simulink library "lib_all"  (Simulink 7.6)
settings: Solver=ode45  SolverMode=SingleTasking  StartTime=0.0  StopTime=10.0  RelTol=1e-3
values from neighbouring files (symbols this model uses):
  map_lib_03 = [6.8679 7.0159 7.4571 8.1849 9.1892 10.456 11.97 13.71 15.655 17.781 20.063 22.474 ... (803 elements, 73x11)]    [scr_simdata.mat]    (also in scr_simdata_all.mat)
  map_lib_06 = [2.5784 2.6727 2.9588 3.4453 4.1444 5.068 6.226 7.6242 9.2634 11.139 13.24 15.552 ... (803 elements, 73x11)]    [scr_simdata.mat]    (also in scr_simdata_all.mat)
  map_lib_09 = [0.0025784 0.0026727 0.0029588 0.0034453 0.0041444 0.005068 0.006226 0.0076242 0.0092634 0.011139 0.01324 0.015552 ... (803 elements, 73x11)]    [scr_simdata.mat]    (also in scr_simdata_all.mat)
  map_lib_10 = [2.74 2.75 2.76 2.77 2.78 2.79 2.8 2.81 2.82 2.83 2.84 2.85 ... (81 elements, 1x81)]    [scr_simdata.mat]    (also in scr_simdata_all.mat)
  map_lib_11 = [1.1983 1.2027 1.207 1.2113 1.2156 1.2198 1.2241 1.2283 1.2326 1.2368 1.241 1.2451 ... (81 elements, 1x81)]    [scr_simdata.mat]    (also in scr_simdata_all.mat)
  map_lib_12 = [2.74 2.75 2.76 2.77 2.78 2.79 2.8 2.81 2.82 2.83 2.84 2.85 ... (81 elements, 1x81)]    [scr_simdata.mat]    (also in scr_simdata_all.mat)
  map_lib_13 = [1.74 1.75 1.76 1.77 1.78 1.79 1.8 1.81 1.82 1.83 1.84 1.85 ... (81 elements, 1x81)]    [scr_simdata.mat]    (also in scr_simdata_all.mat)
  map_lib_16 = [0.00048828125 1.6159 1.8962 2.0821 2.2251 2.3426 2.4433 2.5318 2.611 2.683 2.749 2.8101 ... (1419 elements, 129x11)]    [scr_simdata.mat]    (also in scr_simdata_all.mat)
  map_mr2_01 = [0 800 1600 2400 3200 4000]    [scr_simdata.mat]    (also in scr_simdata_all.mat)
  map_mr2_02 = [1.32 1.32 1.33 1.34 1.36 1.38]    [scr_simdata.mat]    (also in scr_simdata_all.mat)
  map_mr2_03 = [0 800 1600 2400 3200 4000]    [scr_simdata.mat]    (also in scr_simdata_all.mat)
  map_mr2_04 = [1.32 1.32 1.33 1.34 1.36 1.38]    [scr_simdata.mat]    (also in scr_simdata_all.mat)
  map_mr2_05 = [0 800 1600 2400 3200 4000]    [scr_simdata.mat]    (also in scr_simdata_all.mat)
  map_mr2_06 = [1.32 1.32 1.33 1.34 1.36 1.38]    [scr_simdata.mat]    (also in scr_simdata_all.mat)
  Parameter1 = 6    [scr_simdata.mat]    (also in scr_simdata_all.mat)
  Parameter101 = 30    [scr_simdata.mat]    (also in scr_simdata_all.mat)
  Parameter102 = 12    [scr_simdata.mat]    (also in scr_simdata_all.mat)
  Parameter103 = -240    [scr_simdata.mat]    (also in scr_simdata_all.mat)
  Parameter108 = 48    [scr_simdata.mat]    (also in scr_simdata_all.mat)
  Parameter120 = 16    [scr_simdata.mat]    (also in scr_simdata_all.mat)
  Parameter121 = 87    [scr_simdata.mat]    (also in scr_simdata_all.mat)
  Parameter123 = 78    [scr_simdata.mat]    (also in scr_simdata_all.mat)
  Parameter124 = 60    [scr_simdata.mat]    (also in scr_simdata_all.mat)
  Parameter125 = -480    [scr_simdata.mat]    (also in scr_simdata_all.mat)
  Parameter130 = 96    [scr_simdata.mat]    (also in scr_simdata_all.mat)
  Parameter131 = 3    [scr_simdata.mat]    (also in scr_simdata_all.mat)
  Parameter144 = 3    [scr_simdata.mat]    (also in scr_simdata_all.mat)
  Parameter18 = 128    [scr_simdata.mat]    (also in scr_simdata_all.mat)
  Parameter19 = 16    [scr_simdata.mat]    (also in scr_simdata_all.mat)
  Parameter2 = 12    [scr_simdata.mat]    (also in scr_simdata_all.mat)
  Parameter20 = 7    [scr_simdata.mat]    (also in scr_simdata_all.mat)
  Parameter21 = 4    [scr_simdata.mat]    (also in scr_simdata_all.mat)
  Parameter3 = 18    [scr_simdata.mat]    (also in scr_simdata_all.mat)
  Parameter32 = 1    [scr_simdata.mat]    (also in scr_simdata_all.mat)
  Parameter34 = 24    [scr_simdata.mat]    (also in scr_simdata_all.mat)
  Parameter4 = 138    [scr_simdata.mat]    (also in scr_simdata_all.mat)
  Parameter42 = 48    [scr_simdata.mat]    (also in scr_simdata_all.mat)
  Parameter5 = 135    [scr_simdata.mat]    (also in scr_simdata_all.mat)
  Parameter55 = 0    [scr_simdata.mat]    (also in scr_simdata_all.mat)
  Parameter56 = 0    [scr_simdata.mat]    (also in scr_simdata_all.mat)
  Parameter57 = 1    [scr_simdata.mat]    (also in scr_simdata_all.mat)
  Parameter58 = 1    [scr_simdata.mat]    (also in scr_simdata_all.mat)
  Parameter59 = 2    [scr_simdata.mat]    (also in scr_simdata_all.mat)
  Parameter6 = 132    [scr_simdata.mat]    (also in scr_simdata_all.mat)
  Parameter60 = 2    [scr_simdata.mat]    (also in scr_simdata_all.mat)
  Parameter63 = 144    [scr_simdata.mat]    (also in scr_simdata_all.mat)
  Parameter7 = 126    [scr_simdata.mat]    (also in scr_simdata_all.mat)
  Parameter76 = 64    [scr_simdata.mat]    (also in scr_simdata_all.mat)
  Parameter77 = 135    [scr_simdata.mat]    (also in scr_simdata_all.mat)
  Parameter79 = 126    [scr_simdata.mat]    (also in scr_simdata_all.mat)
  Parameter80 = 108    [scr_simdata.mat]    (also in scr_simdata_all.mat)
  Parameter81 = 0    [scr_simdata.mat]    (also in scr_simdata_all.mat)
  Parameter86 = 0    [scr_simdata.mat]    (also in scr_simdata_all.mat)
  Parameter98 = 112    [scr_simdata.mat]    (also in scr_simdata_all.mat)
  Parameter99 = 39    [scr_simdata.mat]    (also in scr_simdata_all.mat)
undefined symbols (not defined in the file or in its folder): cylvolkp_hih_map2h, cylvolkp_map2h, pk1k_map2h
libraries: simulink x2
(part 16 of 36)

== system lib_all/SubSystem800/SubSystem887/SubSystem914/SubSystem922 ==
  note: Text165
  note: Text164
  note: Text163
  Block2387 : Inport
  Block2388 : Inport  Port=2
  Block2389 : Inport  Port=3
  Block2390 : Constant  Value=0  OutDataTypeStr=double  SampleTime=-1
  Block2391 : Constant  OutDataTypeStr=double  SampleTime=-1
  Block2392 : Constant  OutDataTypeStr=double  SampleTime=-1
  Block2393 : Constant  Value=0  OutDataTypeStr=double  SampleTime=-1
  Block2394 : Constant  OutDataTypeStr=double  SampleTime=-1
  Block2395 : Constant  Value=143  OutDataTypeStr=double  SampleTime=-1
  Block2396 : Demux  Outputs=2
  Block2397 : Logic  Operator=NOT
  Block2398 : Mux  Inputs=2
  Block2399 : Mux  Inputs=2
  Block2400 : Sum  OutDataTypeStr=double  RndMeth=Zero  SaturateOnIntegerOverflow=on  InputSameDT=on
  Block2401 : Sum  OutDataTypeStr=double  RndMeth=Zero  SaturateOnIntegerOverflow=on  InputSameDT=on
  Block2402 : Switch  Threshold=0.5  RndMeth=Zero  SaturateOnIntegerOverflow=on  InputSameDT=on
  Block2403 : Switch  Threshold=0.5  RndMeth=Zero  SaturateOnIntegerOverflow=on  InputSameDT=on
  Block2404 : Switch  Threshold=0.5  RndMeth=Zero  SaturateOnIntegerOverflow=on  InputSameDT=on
  SubSystem923 : Subsystem
  SubSystem924 : Subsystem
  SubSystem925 : Subsystem
  SubSystem926 : Subsystem  TreatAsAtomicUnit=on
  SubSystem935 : Subsystem  TreatAsAtomicUnit=on
  SubSystem945 : Subsystem  TreatAsAtomicUnit=on
  Block2449 : Outport
  Block2450 : Outport  Port=2
  wires:
  Block2387/1 -> SubSystem923/1(In1), Block2400/2, Block2398/1
  Block2395/1 -> SubSystem923/2(In2), Block2399/1
  Block2388/1 -> SubSystem924/1(In1), SubSystem925/1(In1)
  Block2390/1 -> SubSystem924/2(In2)
  SubSystem924/1(Out1) -> Block2402/2, SubSystem926/Enable
  SubSystem926/1(Block2418) -> Block2402/1
  Block2391/1 -> SubSystem925/2(In2)
  SubSystem925/1(Out1) -> Block2403/2, SubSystem935/Enable, Block2397/1
  SubSystem935/1(Block2433) -> Block2403/1
  Block2397/1 -> SubSystem945/Enable
  Block2403/1 -> Block2402/3
  SubSystem945/1(Block2448) -> Block2403/3
  Block2396/1 -> Block2449/1
  Block2396/2 -> Block2450/1
  Block2402/1 -> Block2396/1
  SubSystem923/1(Out1) -> Block2404/2
  Block2392/1 -> Block2400/1
  Block2393/1 -> Block2399/2
  Block2399/1 -> Block2404/3
  Block2400/1 -> Block2398/2
  Block2398/1 -> Block2404/1
  Block2389/1 -> SubSystem926/2(Block2406), SubSystem935/2(Block2420), SubSystem945/2(Block2435)
  Block2404/1 -> Block2401/2
  Block2394/1 -> Block2401/1
  Block2401/1 -> SubSystem926/1(Block2405), SubSystem935/1(Block2419), SubSystem945/1(Block2434)

== system lib_all/SubSystem959/SubSystem963/SubSystem1018/SubSystem1019 ==
  note: ***編集禁止***
  In1 : Inport
  In2 : Inport  Port=2
  LO1 : Logic  Operator=OR
  RO1 : RelationalOperator  InputSameDT=on
  RO2 : RelationalOperator  Operator===  InputSameDT=on
  single1 : DataTypeConversion  OutDataTypeStr=single  SaturateOnIntegerOverflow=on  RndMeth=Zero
  single2 : DataTypeConversion  OutDataTypeStr=single  SaturateOnIntegerOverflow=on  RndMeth=Zero
  Out1 : Outport
  wires:
  In2/1 -> single2/1
  In1/1 -> single1/1
  single1/1 -> RO1/1, RO2/1
  single2/1 -> RO1/2, RO2/2
  RO1/1 -> LO1/1
  RO2/1 -> LO1/2
  LO1/1 -> Out1/1

== system lib_all/SubSystem959/SubSystem963/SubSystem1018/SubSystem1020 ==
  note: ***編集禁止***
  In1 : Inport
  In2 : Inport  Port=2
  LO1 : Logic
  RO1 : RelationalOperator  Operator=<  InputSameDT=on
  RO2 : RelationalOperator  Operator=~=  InputSameDT=on
  single1 : DataTypeConversion  OutDataTypeStr=single  SaturateOnIntegerOverflow=on  RndMeth=Zero
  single2 : DataTypeConversion  OutDataTypeStr=single  SaturateOnIntegerOverflow=on  RndMeth=Zero
  Out1 : Outport
  wires:
  In2/1 -> single2/1
  In1/1 -> single1/1
  single1/1 -> RO1/1, RO2/1
  single2/1 -> RO1/2, RO2/2
  RO1/1 -> LO1/1
  RO2/1 -> LO1/2
  LO1/1 -> Out1/1

== system lib_all/SubSystem959/SubSystem963/SubSystem965/SubSystem967 ==
  note: ***編集禁止***
  In1 : Inport
  In2 : Inport  Port=2
  LO1 : Logic
  RO1 : RelationalOperator  Operator=<  InputSameDT=on
  RO2 : RelationalOperator  Operator=~=  InputSameDT=on
  single1 : DataTypeConversion  OutDataTypeStr=single  SaturateOnIntegerOverflow=on  RndMeth=Zero
  single2 : DataTypeConversion  OutDataTypeStr=single  SaturateOnIntegerOverflow=on  RndMeth=Zero
  Out1 : Outport
  wires:
  LO1/1 -> Out1/1
  RO2/1 -> LO1/2
  RO1/1 -> LO1/1
  single2/1 -> RO2/2, RO1/2
  single1/1 -> RO2/1, RO1/1
  In1/1 -> single1/1
  In2/1 -> single2/1

== system lib_all/SubSystem959/SubSystem963/SubSystem965/SubSystem968 ==
  note: Text181
  note: Text180
  Block2503 : Inport
  Block2504 : Inport  Port=2
  Block2505 : Inport  Port=3
  Block2506 : Constant  Value=720  OutDataTypeStr=double  SampleTime=-1
  Block2507 : Constant  Value=0  OutDataTypeStr=double  SampleTime=-1
  Block2508 : Constant  Value=0  OutDataTypeStr=double  SampleTime=-1
  Block2509 : Constant  Value=5  OutDataTypeStr=double  SampleTime=-1
  Block2510 : DataStoreRead  DataStoreName=DSM50
  Block2511 : DataStoreRead  DataStoreName=DSM35
  Block2512 : Gain  Gain=5  SaturateOnIntegerOverflow=on  OutDataTypeStr=Inherit: Same as input  ParamDataTypeStr=Inherit: Same as input
      settings: AttributesFormatString=Attribute513
  Block2513 : Gain  Gain=5  SaturateOnIntegerOverflow=on  OutDataTypeStr=Inherit: Same as input  ParamDataTypeStr=Inherit: Same as input
      settings: AttributesFormatString=Attribute514
  Block2514 : Gain  Gain=1/5  SaturateOnIntegerOverflow=on  OutDataTypeStr=Inherit: Same as input  ParamDataTypeStr=Inherit: Same as input
      settings: AttributesFormatString=Attribute515
  Block2515 : Product  Inputs=*/  OutDataTypeStr=double  SaturateOnIntegerOverflow=on  RndMeth=Zero  InputSameDT=on
  Block2516 : Rounding
  Block2517 : Rounding
  Block2518 : Sum  Inputs=+-+-  OutDataTypeStr=double  RndMeth=Zero  SaturateOnIntegerOverflow=on  InputSameDT=on
  Block2519 : Sum  Inputs=-+  OutDataTypeStr=double  RndMeth=Zero  SaturateOnIntegerOverflow=on  InputSameDT=on
  SubSystem969 : Subsystem  TreatAsAtomicUnit=on  mask(***ATC.DIVISION***){bd_version=1.01.A, r0=チェック有り, r1=, r2=, r3=, r4=, r5=, r6=, r7=, r8=, r9=, c0=, c1=, c2=, c3=, c4=, c5=, c6=, c7=, c8=, c9=}
      mask prompts: bd_version "Version"
      mask help: これはAutoCからのブロックです．編集禁止．
      settings: OpenFcn=warndlg('変更禁止!');
      mask Help: web(ui_blockhelpmng(gcb));
  SubSystem970 : Subsystem  TreatAsAtomicUnit=on  mask(***ATC.DSRVEC***){bd_version=1.01.A, r0=DSM95, r1=-1, r2=, r3=, r4=, r5=, r6=, r7=, r8=, r9=, c0=, c1=, c2=, c3=, c4=, c5=, c6=, c7=, c8=, c9=}
      mask prompts: bd_version "Version"
      mask help: これはAutoCからのブロックです．編集禁止．
      settings: OpenFcn=warndlg('変更禁止!');
      mask Help: web(ui_blockhelpmng(gcb));
      mask Display: disp('ATC.DSRVEC')
  SubSystem971 : Subsystem
      settings: RTWSystemCode=Inline
  Block2528 : Outport
  Block2529 : Outport  Port=2
  wires:
  Block2511/1 -> SubSystem969/2(div)
  Block2519/1 -> Block2529/1
  Block2512/1 -> Block2519/1
  Block2516/1 -> Block2512/1
  Block2515/1 -> Block2516/1
  Block2509/1 -> Block2515/2
  Block2504/1 -> SubSystem970/1(element)
  Block2508/1 -> SubSystem971/3(Block2522)
  Block2518/1 -> SubSystem971/2(Block2521)  "SigLabel84"
  Block2506/1 -> SubSystem971/1(Block2520)
  Block2505/1 -> Block2518/4
  SubSystem971/1(Block2527) -> Block2515/1, Block2519/2, Block2514/1
  Block2503/1 -> Block2513/1
  SubSystem969/1(Out) -> Block2518/3  "SigLabel83"
  Block2507/1 -> SubSystem969/3(init)
  SubSystem970/1(Output) -> SubSystem969/1(mult)
  Block2510/1 -> Block2518/2
  Block2513/1 -> Block2518/1
  Block2514/1 -> Block2517/1
  Block2517/1 -> Block2528/1

== system lib_all/SubSystem959/SubSystem963/SubSystem965/SubSystem974 ==
  note: Text184
  note: Text183
  note: Text182
  Block2530 : Inport
  Block2531 : Inport  Port=2
  Block2532 : Inport  Port=3
  Block2533 : Constant  Value=0  OutDataTypeStr=double  SampleTime=-1
  Block2534 : Constant  OutDataTypeStr=double  SampleTime=-1
  Block2535 : Constant  OutDataTypeStr=double  SampleTime=-1
  Block2536 : Constant  Value=0  OutDataTypeStr=double  SampleTime=-1
  Block2537 : Constant  Value=2  OutDataTypeStr=double  SampleTime=-1
  Block2538 : Constant  OutDataTypeStr=double  SampleTime=-1
  Block2539 : Constant  Value=143  OutDataTypeStr=double  SampleTime=-1
  Block2540 : Demux  Outputs=2
  Block2541 : Logic  Operator=NOT
  Block2542 : Mux  Inputs=2
  Block2543 : Mux  Inputs=2
  Block2544 : Sum  OutDataTypeStr=double  RndMeth=Zero  SaturateOnIntegerOverflow=on  InputSameDT=on
  Block2545 : Sum  OutDataTypeStr=double  RndMeth=Zero  SaturateOnIntegerOverflow=on  InputSameDT=on
  Block{2546..2549} (4 alike) : Switch  Threshold=0.5  RndMeth=Zero  SaturateOnIntegerOverflow=on  InputSameDT=on
  SubSystem1008 : Subsystem  TreatAsAtomicUnit=on
  SubSystem975 : Subsystem
  SubSystem976 : Subsystem
  SubSystem977 : Subsystem
  SubSystem978 : Subsystem
  SubSystem979 : Subsystem  TreatAsAtomicUnit=on
  SubSystem988 : Subsystem  TreatAsAtomicUnit=on
  SubSystem998 : Subsystem  TreatAsAtomicUnit=on
  Block2609 : Outport
  Block2610 : Outport  Port=2
  wires:
  Block2532/1 -> SubSystem1008/2(Block2595), SubSystem998/2(Block2580), SubSystem988/2(Block2565), SubSystem979/2(Block2551)
  Block2549/1 -> Block2547/3
  SubSystem1008/1(Block2608) -> Block2549/3
  SubSystem998/1(Block2593) -> Block2549/1
  Block2541/1 -> SubSystem1008/Enable
  SubSystem978/1(Out1) -> Block2549/2, SubSystem998/Enable, Block2541/1
  Block2537/1 -> SubSystem978/2(In2)
  Block2542/1 -> Block2548/1
  Block2544/1 -> Block2542/2
  Block2543/1 -> Block2548/3
  Block2536/1 -> Block2543/2
  Block2535/1 -> Block2544/1
  SubSystem975/1(Out1) -> Block2548/2
  Block2546/1 -> Block2540/1
  Block2540/2 -> Block2610/1
  Block2540/1 -> Block2609/1
  Block2545/1 -> SubSystem1008/1(Block2594), SubSystem998/1(Block2579), SubSystem988/1(Block2564), SubSystem979/1(Block2550)
  Block2547/1 -> Block2546/3
  SubSystem988/1(Block2578) -> Block2547/1
  SubSystem977/1(Out1) -> SubSystem988/Enable, Block2547/2
  Block2534/1 -> SubSystem977/2(In2)
  SubSystem979/1(Block2563) -> Block2546/1
  SubSystem976/1(Out1) -> SubSystem979/Enable, Block2546/2
  Block2533/1 -> SubSystem976/2(In2)
  Block2531/1 -> SubSystem978/1(In1), SubSystem977/1(In1), SubSystem976/1(In1)
  Block2539/1 -> Block2543/1, SubSystem975/2(In2)
  Block2530/1 -> Block2542/1, Block2544/2, SubSystem975/1(In1)
  Block2538/1 -> Block2545/1
  Block2548/1 -> Block2545/2

== system lib_all/SubSystem1023/SubSystem1025/SubSystem1038/SubSystem1041/SubSystem1042 ==
  Block2712 : Inport
  Block2713 : Inport  Port=2
  Block2714 : Constant  Value=Parameter32  OutDataTypeStr=double  SampleTime=-1
  Block2715 : Constant  Value=2  OutDataTypeStr=double  SampleTime=-1
  Block2716 : Constant  Value=Parameter42  OutDataTypeStr=double  SampleTime=-1
  Block2717 : Constant  Value=0  OutDataTypeStr=double  SampleTime=-1
  Block2718 : Constant  Value=72  OutDataTypeStr=double  SampleTime=-1
  Block2719 : Constant  Value=Parameter34  OutDataTypeStr=double  SampleTime=-1
  Block2720 : Logic  Operator=OR
  Block2721 : Sum  Inputs=+-  OutDataTypeStr=double  RndMeth=Zero  SaturateOnIntegerOverflow=on  InputSameDT=on
  Block2722 : Switch  Threshold=0.5  RndMeth=Zero  SaturateOnIntegerOverflow=on  InputSameDT=on
  Block2723 : Switch  Threshold=0.5  RndMeth=Zero  SaturateOnIntegerOverflow=on  InputSameDT=on
  SubSystem1043 : Subsystem
  SubSystem1044 : Subsystem
  SubSystem1045 : Subsystem
      settings: RTWSystemCode=Inline
  Block2730 : Outport
  wires:
  SubSystem1045/1(Block2729) -> Block2723/1
  Block2718/1 -> SubSystem1045/1(Block2724)
  Block2717/1 -> SubSystem1045/3(Block2726)
  Block2721/1 -> SubSystem1045/2(Block2725)
  Block2712/1 -> Block2723/3, Block2721/1
  Block2713/1 -> SubSystem1043/2(In2), SubSystem1044/1(In1)
  Block2714/1 -> SubSystem1043/1(In1)
  Block2715/1 -> SubSystem1044/2(In2)
  SubSystem1043/1(Out1) -> Block2720/1, Block2722/2
  SubSystem1044/1(Out1) -> Block2720/2
  Block2720/1 -> Block2723/2
  Block2716/1 -> Block2722/1
  Block2719/1 -> Block2722/3
  Block2722/1 -> Block2721/2
  Block2723/1 -> Block2730/1

== system lib_all/SubSystem1023/SubSystem1025/SubSystem1038/SubSystem1041/SubSystem1047 ==
  Block2731 : Inport
  Block2732 : Inport  Port=2
  Block2733 : Inport  Port=3
  Block2734 : Sum  OutDataTypeStr=double  RndMeth=Zero  SaturateOnIntegerOverflow=on  InputSameDT=on
  Block2735 : Switch  Threshold=0.5  RndMeth=Zero  SaturateOnIntegerOverflow=on  InputSameDT=on
  SubSystem1048 : Subsystem
  Block2736 : Outport
  wires:
  Block2732/1 -> SubSystem1048/1(In1), Block2735/3, Block2734/2
  Block2733/1 -> SubSystem1048/2(In2)
  SubSystem1048/1(Out1) -> Block2735/2
  Block2734/1 -> Block2735/1
  Block2735/1 -> Block2736/1
  Block2731/1 -> Block2734/1

== system lib_all/SubSystem1023/SubSystem1025/SubSystem1038/SubSystem1050/SubSystem1051 ==
  note: ***編集禁止***
  In1 : Inport
  In2 : Inport  Port=2
  RO1 : RelationalOperator  Operator=>  InputSameDT=on
  single1 : DataTypeConversion  OutDataTypeStr=single  SaturateOnIntegerOverflow=on  RndMeth=Zero
  single2 : DataTypeConversion  OutDataTypeStr=single  SaturateOnIntegerOverflow=on  RndMeth=Zero
  Out1 : Outport
  wires:
  single2/1 -> RO1/2
  single1/1 -> RO1/1
  In1/1 -> single1/1
  In2/1 -> single2/1
  RO1/1 -> Out1/1

== system lib_all/SubSystem1023/SubSystem1025/SubSystem1052/SubSystem1054/SubSystem1055 ==
  note: ***編集禁止***
  mult : Inport
  div : Inport  Port=2
  init : Inport  Port=3
  Product : Subsystem  TreatAsAtomicUnit=on
      settings: RTWSystemCode=Inline
  Out : Outport
  wires:
  init/1 -> Product/3(init)
  div/1 -> Product/2(div)
  mult/1 -> Product/1(mult)
  Product/1(Out) -> Out/1

== system lib_all/SubSystem1023/SubSystem1025/SubSystem1052/SubSystem1054/SubSystem1056 ==
  note: ***編集禁止***
  element : Inport
  Inside_DSR : DataStoreRead  DataStoreName=DSM95
  Selector : Selector  IndexOptions=Index vector (port)  Indices=[1 3]  OutputSizes=1
  Output : Outport
  wires:
  Inside_DSR/1 -> Selector/1
  element/1 -> Selector/2
  Selector/1 -> Output/1

== system lib_all/SubSystem1023/SubSystem1025/SubSystem1052/SubSystem1054/SubSystem1057 ==
  Block2779 : Inport
  Block2780 : Inport  Port=2
  Block2781 : Inport  Port=3
  Block2782 : Sum  OutDataTypeStr=double  RndMeth=Zero  SaturateOnIntegerOverflow=on  InputSameDT=on
  Block2783 : Sum  Inputs=+-  OutDataTypeStr=double  RndMeth=Zero  SaturateOnIntegerOverflow=on  InputSameDT=on
  Block2784 : Switch  Threshold=0.5  RndMeth=Zero  SaturateOnIntegerOverflow=on  InputSameDT=on
  Block2785 : Switch  Threshold=0.5  RndMeth=Zero  SaturateOnIntegerOverflow=on  InputSameDT=on
  SubSystem1058 : Subsystem
  SubSystem1059 : Subsystem
  Block2786 : Outport
  wires:
  Block2783/1 -> Block2784/1
  Block2784/1 -> Block2786/1
  Block2782/1 -> Block2785/1
  Block2785/1 -> Block2784/3
  SubSystem1059/1(Out1) -> Block2785/2
  Block2781/1 -> SubSystem1059/2(In2)
  SubSystem1058/1(Out1) -> Block2784/2
  Block2779/1 -> SubSystem1058/2(In2), Block2783/2, Block2782/1
  Block2780/1 -> Block2782/2, Block2785/3, SubSystem1059/1(In1), Block2783/1, SubSystem1058/1(In1)

== system lib_all/SubSystem1023/SubSystem1025/SubSystem1052/SubSystem1060/SubSystem1061 ==
  note: ***編集禁止***
  In1 : Inport
  In2 : Inport  Port=2
  LO1 : Logic
  RO1 : RelationalOperator  Operator=<  InputSameDT=on
  RO2 : RelationalOperator  Operator=~=  InputSameDT=on
  single1 : DataTypeConversion  OutDataTypeStr=single  SaturateOnIntegerOverflow=on  RndMeth=Zero
  single2 : DataTypeConversion  OutDataTypeStr=single  SaturateOnIntegerOverflow=on  RndMeth=Zero
  Out1 : Outport
  wires:
  single2/1 -> RO1/2, RO2/2
  single1/1 -> RO1/1, RO2/1
  In1/1 -> single1/1
  In2/1 -> single2/1
  RO1/1 -> LO1/1
  RO2/1 -> LO1/2
  LO1/1 -> Out1/1

== system lib_all/SubSystem1023/SubSystem1025/SubSystem1052/SubSystem1060/SubSystem1062 ==
  note: ***編集禁止***
  In1 : Inport
  In2 : Inport  Port=2
  RO1 : RelationalOperator  Operator===  InputSameDT=on
  single1 : DataTypeConversion  OutDataTypeStr=single  SaturateOnIntegerOverflow=on  RndMeth=Zero
  single2 : DataTypeConversion  OutDataTypeStr=single  SaturateOnIntegerOverflow=on  RndMeth=Zero
  Out1 : Outport
  wires:
  single2/1 -> RO1/2
  single1/1 -> RO1/1
  In1/1 -> single1/1
  In2/1 -> single2/1
  RO1/1 -> Out1/1

== system lib_all/SubSystem1023/SubSystem1025/SubSystem1052/SubSystem1060/SubSystem1063 ==
  note: ***編集禁止***
  In1 : Inport
  In2 : Inport  Port=2
  RO1 : RelationalOperator  Operator===  InputSameDT=on
  single1 : DataTypeConversion  OutDataTypeStr=single  SaturateOnIntegerOverflow=on  RndMeth=Zero
  single2 : DataTypeConversion  OutDataTypeStr=single  SaturateOnIntegerOverflow=on  RndMeth=Zero
  Out1 : Outport
  wires:
  single2/1 -> RO1/2
  single1/1 -> RO1/1
  In1/1 -> single1/1
  In2/1 -> single2/1
  RO1/1 -> Out1/1

== system lib_all/SubSystem1023/SubSystem1025/SubSystem1052/SubSystem1060/SubSystem1064 ==
  Block2807 : Inport
  Block2808 : Inport  Port=2
  Block2809 : EnablePort
  Block2810 : Demux  Outputs=2
  Block2811 : Mux  Inputs=2
  SubSystem1065 : Subsystem
  SubSystem1069 : Subsystem
  Block2820 : Outport
  wires:
  Block2811/1 -> Block2820/1
  SubSystem1069/1(Block2819) -> Block2811/2
  SubSystem1065/1(Block2815) -> Block2811/1
  Block2810/2 -> SubSystem1069/2(Block2817)
  Block2810/1 -> SubSystem1065/2(Block2813)
  Block2808/1 -> SubSystem1065/1(Block2812), SubSystem1069/1(Block2816)
  Block2807/1 -> Block2810/1

== system lib_all/SubSystem1023/SubSystem1025/SubSystem1052/SubSystem1060/SubSystem1073 ==
  Block2821 : Inport
  Block2822 : Inport  Port=2
  Block2823 : EnablePort
  Block2824 : Demux  Outputs=2
  Block2825 : Mux  Inputs=2
  Block2826 : Sum  OutDataTypeStr=double  RndMeth=Zero  SaturateOnIntegerOverflow=on  InputSameDT=on
  SubSystem1074 : Subsystem  TreatAsAtomicUnit=on  mask(***ATC.DSRVEC***){bd_version=1.01.A, r0=DSM4, r1=-1, r2=, r3=, r4=, r5=, r6=, r7=, r8=, r9=, c0=, c1=, c2=, c3=, c4=, c5=, c6=, c7=, c8=, c9=}
      mask prompts: bd_version "Version"
      mask help: これはAutoCからのブロックです．編集禁止．
      settings: OpenFcn=warndlg('変更禁止!');
      mask Help: web(ui_blockhelpmng(gcb));
      mask Display: disp('ATC.DSRVEC')
  SubSystem1075 : Subsystem
  SubSystem1079 : Subsystem
  Block2835 : Outport
  wires:
  Block2822/1 -> SubSystem1074/1(element), SubSystem1075/1(Block2827), SubSystem1079/1(Block2831)
  Block2825/1 -> Block2826/2
  SubSystem1074/1(Output) -> Block2826/1
  Block2826/1 -> Block2835/1
  SubSystem1079/1(Block2834) -> Block2825/2
  SubSystem1075/1(Block2830) -> Block2825/1
  Block2821/1 -> Block2824/1
  Block2824/1 -> SubSystem1075/2(Block2828)
  Block2824/2 -> SubSystem1079/2(Block2832)

== system lib_all/SubSystem1023/SubSystem1025/SubSystem1052/SubSystem1060/SubSystem1083 ==
  Block2836 : Inport
  Block2837 : Inport  Port=2
  Block2838 : EnablePort
  Block2839 : Demux  Outputs=2
  Block2840 : Mux  Inputs=2
  Block2841 : Sum  OutDataTypeStr=double  RndMeth=Zero  SaturateOnIntegerOverflow=on  InputSameDT=on
  SubSystem1084 : Subsystem  TreatAsAtomicUnit=on  mask(***ATC.DSRVEC***){bd_version=1.01.A, r0=DSM2, r1=-1, r2=, r3=, r4=, r5=, r6=, r7=, r8=, r9=, c0=, c1=, c2=, c3=, c4=, c5=, c6=, c7=, c8=, c9=}
      mask prompts: bd_version "Version"
      mask help: これはAutoCからのブロックです．編集禁止．
      settings: OpenFcn=warndlg('変更禁止!');
      mask Help: web(ui_blockhelpmng(gcb));
      mask Display: disp('ATC.DSRVEC')
  SubSystem1085 : Subsystem
  SubSystem1089 : Subsystem
  Block2850 : Outport
  wires:
  SubSystem1084/1(Output) -> Block2841/1
  Block2837/1 -> SubSystem1084/1(element), SubSystem1085/1(Block2842), SubSystem1089/1(Block2846)
  Block2840/1 -> Block2841/2
  Block2841/1 -> Block2850/1
  SubSystem1089/1(Block2849) -> Block2840/2
  SubSystem1085/1(Block2845) -> Block2840/1
  Block2839/2 -> SubSystem1089/2(Block2847)
  Block2839/1 -> SubSystem1085/2(Block2843)
  Block2836/1 -> Block2839/1

== system lib_all/SubSystem1023/SubSystem1093/SubSystem1106/SubSystem1109/SubSystem1110 ==
  Block2937 : Inport
  Block2938 : Inport  Port=2
  Block2939 : Constant  Value=Parameter32  OutDataTypeStr=double  SampleTime=-1
  Block2940 : Constant  Value=2  OutDataTypeStr=double  SampleTime=-1
  Block2941 : Constant  Value=Parameter42  OutDataTypeStr=double  SampleTime=-1
  Block2942 : Constant  Value=0  OutDataTypeStr=double  SampleTime=-1
  Block2943 : Constant  Value=72  OutDataTypeStr=double  SampleTime=-1
  Block2944 : Constant  Value=Parameter34  OutDataTypeStr=double  SampleTime=-1
  Block2945 : Logic  Operator=OR
  Block2946 : Sum  Inputs=+-  OutDataTypeStr=double  RndMeth=Zero  SaturateOnIntegerOverflow=on  InputSameDT=on
  Block2947 : Switch  Threshold=0.5  RndMeth=Zero  SaturateOnIntegerOverflow=on  InputSameDT=on
  Block2948 : Switch  Threshold=0.5  RndMeth=Zero  SaturateOnIntegerOverflow=on  InputSameDT=on
  SubSystem1111 : Subsystem
  SubSystem1112 : Subsystem
  SubSystem1113 : Subsystem
      settings: RTWSystemCode=Inline
  Block2955 : Outport
  wires:
  SubSystem1113/1(Block2954) -> Block2948/1
  Block2943/1 -> SubSystem1113/1(Block2949)
  Block2942/1 -> SubSystem1113/3(Block2951)
  Block2946/1 -> SubSystem1113/2(Block2950)
  Block2937/1 -> Block2948/3, Block2946/1
  Block2938/1 -> SubSystem1111/2(In2), SubSystem1112/1(In1)
  Block2939/1 -> SubSystem1111/1(In1)
  Block2940/1 -> SubSystem1112/2(In2)
  SubSystem1111/1(Out1) -> Block2945/1, Block2947/2
  SubSystem1112/1(Out1) -> Block2945/2
  Block2945/1 -> Block2948/2
  Block2941/1 -> Block2947/1
  Block2944/1 -> Block2947/3
  Block2947/1 -> Block2946/2
  Block2948/1 -> Block2955/1

== system lib_all/SubSystem1023/SubSystem1093/SubSystem1106/SubSystem1109/SubSystem1115 ==
  Block2956 : Inport
  Block2957 : Inport  Port=2
  Block2958 : Inport  Port=3
  Block2959 : Sum  OutDataTypeStr=double  RndMeth=Zero  SaturateOnIntegerOverflow=on  InputSameDT=on
  Block2960 : Switch  Threshold=0.5  RndMeth=Zero  SaturateOnIntegerOverflow=on  InputSameDT=on
  SubSystem1116 : Subsystem
  Block2961 : Outport
  wires:
  Block2957/1 -> SubSystem1116/1(In1), Block2960/3, Block2959/2
  Block2958/1 -> SubSystem1116/2(In2)
  SubSystem1116/1(Out1) -> Block2960/2
  Block2959/1 -> Block2960/1
  Block2960/1 -> Block2961/1
  Block2956/1 -> Block2959/1

== system lib_all/SubSystem1023/SubSystem1093/SubSystem1106/SubSystem1118/SubSystem1119 ==
  note: ***編集禁止***
  In1 : Inport
  In2 : Inport  Port=2
  RO1 : RelationalOperator  Operator=>  InputSameDT=on
  single1 : DataTypeConversion  OutDataTypeStr=single  SaturateOnIntegerOverflow=on  RndMeth=Zero
  single2 : DataTypeConversion  OutDataTypeStr=single  SaturateOnIntegerOverflow=on  RndMeth=Zero
  Out1 : Outport
  wires:
  single2/1 -> RO1/2
  single1/1 -> RO1/1
  In1/1 -> single1/1
  In2/1 -> single2/1
  RO1/1 -> Out1/1

== system lib_all/SubSystem1023/SubSystem1093/SubSystem1120/SubSystem1122/SubSystem1123 ==
  note: ***編集禁止***
  mult : Inport
  div : Inport  Port=2
  init : Inport  Port=3
  Product : Subsystem  TreatAsAtomicUnit=on
      settings: RTWSystemCode=Inline
  Out : Outport
  wires:
  init/1 -> Product/3(init)
  div/1 -> Product/2(div)
  mult/1 -> Product/1(mult)
  Product/1(Out) -> Out/1

== system lib_all/SubSystem1023/SubSystem1093/SubSystem1120/SubSystem1122/SubSystem1124 ==
  note: ***編集禁止***
  element : Inport
  Inside_DSR : DataStoreRead  DataStoreName=DSM95
  Selector : Selector  IndexOptions=Index vector (port)  Indices=[1 3]  OutputSizes=1
  Output : Outport
  wires:
  Inside_DSR/1 -> Selector/1
  element/1 -> Selector/2
  Selector/1 -> Output/1

== system lib_all/SubSystem1023/SubSystem1093/SubSystem1120/SubSystem1122/SubSystem1125 ==
  Block3004 : Inport
  Block3005 : Inport  Port=2
  Block3006 : Inport  Port=3
  Block3007 : Sum  OutDataTypeStr=double  RndMeth=Zero  SaturateOnIntegerOverflow=on  InputSameDT=on
  Block3008 : Sum  Inputs=+-  OutDataTypeStr=double  RndMeth=Zero  SaturateOnIntegerOverflow=on  InputSameDT=on
  Block3009 : Switch  Threshold=0.5  RndMeth=Zero  SaturateOnIntegerOverflow=on  InputSameDT=on
  Block3010 : Switch  Threshold=0.5  RndMeth=Zero  SaturateOnIntegerOverflow=on  InputSameDT=on
  SubSystem1126 : Subsystem
  SubSystem1127 : Subsystem
  Block3011 : Outport
  wires:
  Block3008/1 -> Block3009/1
  Block3009/1 -> Block3011/1
  Block3007/1 -> Block3010/1
  Block3010/1 -> Block3009/3
  SubSystem1127/1(Out1) -> Block3010/2
  Block3006/1 -> SubSystem1127/2(In2)
  SubSystem1126/1(Out1) -> Block3009/2
  Block3004/1 -> SubSystem1126/2(In2), Block3008/2, Block3007/1
  Block3005/1 -> Block3007/2, Block3010/3, SubSystem1127/1(In1), Block3008/1, SubSystem1126/1(In1)

== system lib_all/SubSystem1023/SubSystem1093/SubSystem1120/SubSystem1128/SubSystem1129 ==
  note: ***編集禁止***
  In1 : Inport
  In2 : Inport  Port=2
  LO1 : Logic
  RO1 : RelationalOperator  Operator=<  InputSameDT=on
  RO2 : RelationalOperator  Operator=~=  InputSameDT=on
  single1 : DataTypeConversion  OutDataTypeStr=single  SaturateOnIntegerOverflow=on  RndMeth=Zero
  single2 : DataTypeConversion  OutDataTypeStr=single  SaturateOnIntegerOverflow=on  RndMeth=Zero
  Out1 : Outport
  wires:
  single2/1 -> RO1/2, RO2/2
  single1/1 -> RO1/1, RO2/1
  In1/1 -> single1/1
  In2/1 -> single2/1
  RO1/1 -> LO1/1
  RO2/1 -> LO1/2
  LO1/1 -> Out1/1

== system lib_all/SubSystem1023/SubSystem1093/SubSystem1120/SubSystem1128/SubSystem1130 ==
  note: ***編集禁止***
  In1 : Inport
  In2 : Inport  Port=2
  RO1 : RelationalOperator  Operator===  InputSameDT=on
  single1 : DataTypeConversion  OutDataTypeStr=single  SaturateOnIntegerOverflow=on  RndMeth=Zero
  single2 : DataTypeConversion  OutDataTypeStr=single  SaturateOnIntegerOverflow=on  RndMeth=Zero
  Out1 : Outport
  wires:
  single2/1 -> RO1/2
  single1/1 -> RO1/1
  In1/1 -> single1/1
  In2/1 -> single2/1
  RO1/1 -> Out1/1

== system lib_all/SubSystem1023/SubSystem1093/SubSystem1120/SubSystem1128/SubSystem1131 ==
  note: ***編集禁止***
  In1 : Inport
  In2 : Inport  Port=2
  RO1 : RelationalOperator  Operator===  InputSameDT=on
  single1 : DataTypeConversion  OutDataTypeStr=single  SaturateOnIntegerOverflow=on  RndMeth=Zero
  single2 : DataTypeConversion  OutDataTypeStr=single  SaturateOnIntegerOverflow=on  RndMeth=Zero
  Out1 : Outport
  wires:
  single2/1 -> RO1/2
  single1/1 -> RO1/1
  In1/1 -> single1/1
  In2/1 -> single2/1
  RO1/1 -> Out1/1

== system lib_all/SubSystem1023/SubSystem1093/SubSystem1120/SubSystem1128/SubSystem1132 ==
  Block3032 : Inport
  Block3033 : Inport  Port=2
  Block3034 : EnablePort
  Block3035 : Demux  Outputs=2
  Block3036 : Mux  Inputs=2
  SubSystem1133 : Subsystem
  SubSystem1137 : Subsystem
  Block3045 : Outport
  wires:
  Block3036/1 -> Block3045/1
  SubSystem1137/1(Block3044) -> Block3036/2
  SubSystem1133/1(Block3040) -> Block3036/1
  Block3035/2 -> SubSystem1137/2(Block3042)
  Block3035/1 -> SubSystem1133/2(Block3038)
  Block3033/1 -> SubSystem1133/1(Block3037), SubSystem1137/1(Block3041)
  Block3032/1 -> Block3035/1

== system lib_all/SubSystem1023/SubSystem1093/SubSystem1120/SubSystem1128/SubSystem1141 ==
  Block3046 : Inport
  Block3047 : Inport  Port=2
  Block3048 : EnablePort
  Block3049 : Demux  Outputs=2
  Block3050 : Mux  Inputs=2
  Block3051 : Sum  OutDataTypeStr=double  RndMeth=Zero  SaturateOnIntegerOverflow=on  InputSameDT=on
  SubSystem1142 : Subsystem  TreatAsAtomicUnit=on  mask(***ATC.DSRVEC***){bd_version=1.01.A, r0=DSM4, r1=-1, r2=, r3=, r4=, r5=, r6=, r7=, r8=, r9=, c0=, c1=, c2=, c3=, c4=, c5=, c6=, c7=, c8=, c9=}
      mask prompts: bd_version "Version"
      mask help: これはAutoCからのブロックです．編集禁止．
      settings: OpenFcn=warndlg('変更禁止!');
      mask Help: web(ui_blockhelpmng(gcb));
      mask Display: disp('ATC.DSRVEC')
  SubSystem1143 : Subsystem
  SubSystem1147 : Subsystem
  Block3060 : Outport
  wires:
  Block3047/1 -> SubSystem1142/1(element), SubSystem1143/1(Block3052), SubSystem1147/1(Block3056)
  Block3050/1 -> Block3051/2
  SubSystem1142/1(Output) -> Block3051/1
  Block3051/1 -> Block3060/1
  SubSystem1147/1(Block3059) -> Block3050/2
  SubSystem1143/1(Block3055) -> Block3050/1
  Block3046/1 -> Block3049/1
  Block3049/1 -> SubSystem1143/2(Block3053)
  Block3049/2 -> SubSystem1147/2(Block3057)

== system lib_all/SubSystem1023/SubSystem1093/SubSystem1120/SubSystem1128/SubSystem1151 ==
  Block3061 : Inport
  Block3062 : Inport  Port=2
  Block3063 : EnablePort
  Block3064 : Demux  Outputs=2
  Block3065 : Mux  Inputs=2
  Block3066 : Sum  OutDataTypeStr=double  RndMeth=Zero  SaturateOnIntegerOverflow=on  InputSameDT=on
  SubSystem1152 : Subsystem  TreatAsAtomicUnit=on  mask(***ATC.DSRVEC***){bd_version=1.01.A, r0=DSM2, r1=-1, r2=, r3=, r4=, r5=, r6=, r7=, r8=, r9=, c0=, c1=, c2=, c3=, c4=, c5=, c6=, c7=, c8=, c9=}
      mask prompts: bd_version "Version"
      mask help: これはAutoCからのブロックです．編集禁止．
      settings: OpenFcn=warndlg('変更禁止!');
      mask Help: web(ui_blockhelpmng(gcb));
      mask Display: disp('ATC.DSRVEC')
  SubSystem1153 : Subsystem
  SubSystem1157 : Subsystem
  Block3075 : Outport
  wires:
  SubSystem1152/1(Output) -> Block3066/1
  Block3062/1 -> SubSystem1152/1(element), SubSystem1153/1(Block3067), SubSystem1157/1(Block3071)
  Block3065/1 -> Block3066/2
  Block3066/1 -> Block3075/1
  SubSystem1157/1(Block3074) -> Block3065/2
  SubSystem1153/1(Block3070) -> Block3065/1
  Block3064/2 -> SubSystem1157/2(Block3072)
  Block3064/1 -> SubSystem1153/2(Block3068)
  Block3061/1 -> Block3064/1

== system lib_all/SubSystem1023/SubSystem1161/SubSystem1164/SubSystem1165/SubSystem1166 ==
  note: ***編集禁止***
  In1 : Inport
  In2 : Inport  Port=2
  RO1 : RelationalOperator  Operator===  InputSameDT=on
  single1 : DataTypeConversion  OutDataTypeStr=single  SaturateOnIntegerOverflow=on  RndMeth=Zero
  single2 : DataTypeConversion  OutDataTypeStr=single  SaturateOnIntegerOverflow=on  RndMeth=Zero
  Out1 : Outport
  wires:
  RO1/1 -> Out1/1
  In2/1 -> single2/1
  In1/1 -> single1/1
  single1/1 -> RO1/1
  single2/1 -> RO1/2

== system lib_all/SubSystem1023/SubSystem1161/SubSystem1164/SubSystem1165/SubSystem1167 ==
  note: ***編集禁止***
  In1 : Inport
  In2 : Inport  Port=2
  RO1 : RelationalOperator  Operator===  InputSameDT=on
  single1 : DataTypeConversion  OutDataTypeStr=single  SaturateOnIntegerOverflow=on  RndMeth=Zero
  single2 : DataTypeConversion  OutDataTypeStr=single  SaturateOnIntegerOverflow=on  RndMeth=Zero
  Out1 : Outport
  wires:
  RO1/1 -> Out1/1
  In2/1 -> single2/1
  In1/1 -> single1/1
  single1/1 -> RO1/1
  single2/1 -> RO1/2

== system lib_all/SubSystem1023/SubSystem1161/SubSystem1164/SubSystem1165/SubSystem1168 ==
  Block3109 : Inport
  Block3110 : Inport  Port=2
  Block3111 : Inport  Port=3
  Block3112 : Sum  OutDataTypeStr=double  RndMeth=Zero  SaturateOnIntegerOverflow=on  InputSameDT=on
  Block3113 : Switch  Threshold=0.5  RndMeth=Zero  SaturateOnIntegerOverflow=on  InputSameDT=on
  SubSystem1169 : Subsystem
  Block3114 : Outport
  wires:
  Block3109/1 -> Block3112/1
  Block3113/1 -> Block3114/1
  Block3112/1 -> Block3113/1
  SubSystem1169/1(Out1) -> Block3113/2
  Block3111/1 -> SubSystem1169/2(In2)
  Block3110/1 -> Block3112/2, Block3113/3, SubSystem1169/1(In1)

== system lib_all/SubSystem1023/SubSystem1161/SubSystem1164/SubSystem1170/SubSystem1171 ==
  note: ***編集禁止***
  In1 : Inport
  In2 : Inport  Port=2
  LO1 : Logic
  RO1 : RelationalOperator  Operator=<  InputSameDT=on
  RO2 : RelationalOperator  Operator=~=  InputSameDT=on
  single1 : DataTypeConversion  OutDataTypeStr=single  SaturateOnIntegerOverflow=on  RndMeth=Zero
  single2 : DataTypeConversion  OutDataTypeStr=single  SaturateOnIntegerOverflow=on  RndMeth=Zero
  Out1 : Outport
  wires:
  In2/1 -> single2/1
  In1/1 -> single1/1
  single1/1 -> RO1/1, RO2/1
  single2/1 -> RO1/2, RO2/2
  RO1/1 -> LO1/1
  RO2/1 -> LO1/2
  LO1/1 -> Out1/1

== system lib_all/SubSystem1023/SubSystem1175/SubSystem1178/SubSystem1179/SubSystem1180 ==
  note: ***編集禁止***
  In1 : Inport
  In2 : Inport  Port=2
  RO1 : RelationalOperator  Operator===  InputSameDT=on
  single1 : DataTypeConversion  OutDataTypeStr=single  SaturateOnIntegerOverflow=on  RndMeth=Zero
  single2 : DataTypeConversion  OutDataTypeStr=single  SaturateOnIntegerOverflow=on  RndMeth=Zero
  Out1 : Outport
  wires:
  RO1/1 -> Out1/1
  In2/1 -> single2/1
  In1/1 -> single1/1
  single1/1 -> RO1/1
  single2/1 -> RO1/2

== system lib_all/SubSystem1023/SubSystem1175/SubSystem1178/SubSystem1179/SubSystem1181 ==
  note: ***編集禁止***
  In1 : Inport
  In2 : Inport  Port=2
  RO1 : RelationalOperator  Operator===  InputSameDT=on
  single1 : DataTypeConversion  OutDataTypeStr=single  SaturateOnIntegerOverflow=on  RndMeth=Zero
  single2 : DataTypeConversion  OutDataTypeStr=single  SaturateOnIntegerOverflow=on  RndMeth=Zero
  Out1 : Outport
  wires:
  RO1/1 -> Out1/1
  In2/1 -> single2/1
  In1/1 -> single1/1
  single1/1 -> RO1/1
  single2/1 -> RO1/2

== system lib_all/SubSystem1023/SubSystem1175/SubSystem1178/SubSystem1179/SubSystem1182 ==
  Block3168 : Inport
  Block3169 : Inport  Port=2
  Block3170 : Inport  Port=3
  Block3171 : Sum  OutDataTypeStr=double  RndMeth=Zero  SaturateOnIntegerOverflow=on  InputSameDT=on
  Block3172 : Switch  Threshold=0.5  RndMeth=Zero  SaturateOnIntegerOverflow=on  InputSameDT=on
  SubSystem1183 : Subsystem
  Block3173 : Outport
  wires:
  Block3168/1 -> Block3171/1
  Block3172/1 -> Block3173/1
  Block3171/1 -> Block3172/1
  SubSystem1183/1(Out1) -> Block3172/2
  Block3170/1 -> SubSystem1183/2(In2)
  Block3169/1 -> Block3171/2, Block3172/3, SubSystem1183/1(In1)

== system lib_all/SubSystem1023/SubSystem1175/SubSystem1178/SubSystem1184/SubSystem1185 ==
  note: ***編集禁止***
  In1 : Inport
  In2 : Inport  Port=2
  LO1 : Logic
  RO1 : RelationalOperator  Operator=<  InputSameDT=on
  RO2 : RelationalOperator  Operator=~=  InputSameDT=on
  single1 : DataTypeConversion  OutDataTypeStr=single  SaturateOnIntegerOverflow=on  RndMeth=Zero
  single2 : DataTypeConversion  OutDataTypeStr=single  SaturateOnIntegerOverflow=on  RndMeth=Zero
  Out1 : Outport
  wires:
  In2/1 -> single2/1
  In1/1 -> single1/1
  single1/1 -> RO1/1, RO2/1
  single2/1 -> RO1/2, RO2/2
  RO1/1 -> LO1/1
  RO2/1 -> LO1/2
  LO1/1 -> Out1/1

== system lib_all/SubSystem1196/SubSystem1206/SubSystem1208/SubSystem1211/SubSystem1212 ==
  note: ***編集禁止***
  mult : Inport
  div : Inport  Port=2
  init : Inport  Port=3
  Product : Subsystem  TreatAsAtomicUnit=on
      settings: RTWSystemCode=Inline
  Out : Outport
  wires:
  Product/1(Out) -> Out/1
  mult/1 -> Product/1(mult)
  div/1 -> Product/2(div)
  init/1 -> Product/3(init)

== system lib_all/SubSystem1196/SubSystem1206/SubSystem1208/SubSystem1211/SubSystem1213 ==
  note: ***編集禁止***
  element : Inport
  Inside_DSR : DataStoreRead  DataStoreName=DSM95
  Selector : Selector  IndexOptions=Index vector (port)  Indices=[1 3]  OutputSizes=1
  Output : Outport
  wires:
  Selector/1 -> Output/1
  element/1 -> Selector/2
  Inside_DSR/1 -> Selector/1

== system lib_all/SubSystem1196/SubSystem1206/SubSystem1208/SubSystem1211/SubSystem1214 ==
  Block3307 : Inport
  Block3308 : Inport  Port=2
  Block3309 : Inport  Port=3
  Block3310 : Sum  OutDataTypeStr=double  RndMeth=Zero  SaturateOnIntegerOverflow=on  InputSameDT=on
  Block3311 : Sum  Inputs=+-  OutDataTypeStr=double  RndMeth=Zero  SaturateOnIntegerOverflow=on  InputSameDT=on
  Block3312 : Switch  Threshold=0.5  RndMeth=Zero  SaturateOnIntegerOverflow=on  InputSameDT=on
  Block3313 : Switch  Threshold=0.5  RndMeth=Zero  SaturateOnIntegerOverflow=on  InputSameDT=on
  SubSystem1215 : Subsystem
  SubSystem1216 : Subsystem
  Block3314 : Outport
  wires:
  Block3308/1 -> SubSystem1215/1(In1), Block3311/1, SubSystem1216/1(In1), Block3313/3, Block3310/2
  Block3307/1 -> Block3310/1, Block3311/2, SubSystem1215/2(In2)
  SubSystem1215/1(Out1) -> Block3312/2
  Block3309/1 -> SubSystem1216/2(In2)
  SubSystem1216/1(Out1) -> Block3313/2
  Block3313/1 -> Block3312/3
  Block3310/1 -> Block3313/1
  Block3312/1 -> Block3314/1
  Block3311/1 -> Block3312/1

== system lib_all/SubSystem1196/SubSystem1206/SubSystem1208/SubSystem1217/SubSystem1218 ==
  note: ***編集禁止***
  In1 : Inport
  In2 : Inport  Port=2
  LO1 : Logic
  RO1 : RelationalOperator  Operator=<  InputSameDT=on
  RO2 : RelationalOperator  Operator=~=  InputSameDT=on
  single1 : DataTypeConversion  OutDataTypeStr=single  SaturateOnIntegerOverflow=on  RndMeth=Zero
  single2 : DataTypeConversion  OutDataTypeStr=single  SaturateOnIntegerOverflow=on  RndMeth=Zero
  Out1 : Outport
  wires:
  LO1/1 -> Out1/1
  RO2/1 -> LO1/2
  RO1/1 -> LO1/1
  In2/1 -> single2/1
  In1/1 -> single1/1
  single1/1 -> RO2/1, RO1/1
  single2/1 -> RO2/2, RO1/2

== system lib_all/SubSystem1196/SubSystem1206/SubSystem1208/SubSystem1217/SubSystem1219 ==
  note: ***編集禁止***
  In1 : Inport
  In2 : Inport  Port=2
  RO1 : RelationalOperator  Operator===  InputSameDT=on
  single1 : DataTypeConversion  OutDataTypeStr=single  SaturateOnIntegerOverflow=on  RndMeth=Zero
  single2 : DataTypeConversion  OutDataTypeStr=single  SaturateOnIntegerOverflow=on  RndMeth=Zero
  Out1 : Outport
  wires:
  RO1/1 -> Out1/1
  In2/1 -> single2/1
  In1/1 -> single1/1
  single1/1 -> RO1/1
  single2/1 -> RO1/2

== system lib_all/SubSystem1196/SubSystem1206/SubSystem1208/SubSystem1217/SubSystem1220 ==
  note: ***編集禁止***
  In1 : Inport
  In2 : Inport  Port=2
  RO1 : RelationalOperator  Operator===  InputSameDT=on
  single1 : DataTypeConversion  OutDataTypeStr=single  SaturateOnIntegerOverflow=on  RndMeth=Zero
  single2 : DataTypeConversion  OutDataTypeStr=single  SaturateOnIntegerOverflow=on  RndMeth=Zero
  Out1 : Outport
  wires:
  RO1/1 -> Out1/1
  In2/1 -> single2/1
  In1/1 -> single1/1
  single1/1 -> RO1/1
  single2/1 -> RO1/2

== system lib_all/SubSystem1196/SubSystem1206/SubSystem1208/SubSystem1217/SubSystem1221 ==
  note: ***編集禁止***
  In1 : Inport
  In2 : Inport  Port=2
  RO1 : RelationalOperator  Operator===  InputSameDT=on
  single1 : DataTypeConversion  OutDataTypeStr=single  SaturateOnIntegerOverflow=on  RndMeth=Zero
  single2 : DataTypeConversion  OutDataTypeStr=single  SaturateOnIntegerOverflow=on  RndMeth=Zero
  Out1 : Outport
  wires:
  RO1/1 -> Out1/1
  In2/1 -> single2/1
  In1/1 -> single1/1
  single1/1 -> RO1/1
  single2/1 -> RO1/2

== system lib_all/SubSystem1196/SubSystem1206/SubSystem1208/SubSystem1217/SubSystem1222 ==
  Block3337 : Inport
  Block3338 : Inport  Port=2
  Block3339 : EnablePort
  Block3340 : Demux  Outputs=2
  Block3341 : Mux  Inputs=2
  SubSystem1223 : Subsystem
  SubSystem1227 : Subsystem
  Block3350 : Outport
  wires:
  Block3337/1 -> Block3340/1
  Block3338/1 -> SubSystem1227/1(Block3346), SubSystem1223/1(Block3342)
  Block3340/1 -> SubSystem1223/2(Block3343)
  Block3340/2 -> SubSystem1227/2(Block3347)
  SubSystem1223/1(Block3345) -> Block3341/1
  SubSystem1227/1(Block3349) -> Block3341/2
  Block3341/1 -> Block3350/1

== system lib_all/SubSystem1196/SubSystem1206/SubSystem1208/SubSystem1217/SubSystem1231 ==
  Block3351 : Inport
  Block3352 : Inport  Port=2
  Block3353 : EnablePort
  Block3354 : Demux  Outputs=2
  Block3355 : Mux  Inputs=2
  Block3356 : Sum  OutDataTypeStr=double  RndMeth=Zero  SaturateOnIntegerOverflow=on  InputSameDT=on
  SubSystem1232 : Subsystem  TreatAsAtomicUnit=on  mask(***ATC.DSRVEC***){bd_version=1.01.A, r0=DSM1, r1=-1, r2=, r3=, r4=, r5=, r6=, r7=, r8=, r9=, c0=, c1=, c2=, c3=, c4=, c5=, c6=, c7=, c8=, c9=}
      mask prompts: bd_version "Version"
      mask help: これはAutoCからのブロックです．編集禁止．
      settings: OpenFcn=warndlg('変更禁止!');
      mask Help: web(ui_blockhelpmng(gcb));
      mask Display: disp('ATC.DSRVEC')
  SubSystem1233 : Subsystem
  SubSystem1237 : Subsystem
  Block3365 : Outport
  wires:
  Block3351/1 -> Block3354/1
  Block3354/1 -> SubSystem1233/2(Block3358)
  Block3354/2 -> SubSystem1237/2(Block3362)
  SubSystem1233/1(Block3360) -> Block3355/1
  SubSystem1237/1(Block3364) -> Block3355/2
  Block3356/1 -> Block3365/1
  SubSystem1232/1(Output) -> Block3356/1
  Block3355/1 -> Block3356/2
  Block3352/1 -> SubSystem1237/1(Block3361), SubSystem1233/1(Block3357), SubSystem1232/1(element)

== system lib_all/SubSystem1196/SubSystem1206/SubSystem1208/SubSystem1217/SubSystem1241 ==
  Block3366 : Inport
  Block3367 : Inport  Port=2
  Block3368 : EnablePort
  Block3369 : Demux  Outputs=2
  Block3370 : Mux  Inputs=2
  Block3371 : Sum  OutDataTypeStr=double  RndMeth=Zero  SaturateOnIntegerOverflow=on  InputSameDT=on
  SubSystem1242 : Subsystem  TreatAsAtomicUnit=on  mask(***ATC.DSRVEC***){bd_version=1.01.A, r0=DSM5, r1=-1, r2=, r3=, r4=, r5=, r6=, r7=, r8=, r9=, c0=, c1=, c2=, c3=, c4=, c5=, c6=, c7=, c8=, c9=}
      mask prompts: bd_version "Version"
      mask help: これはAutoCからのブロックです．編集禁止．
      settings: OpenFcn=warndlg('変更禁止!');
      mask Help: web(ui_blockhelpmng(gcb));
      mask Display: disp('ATC.DSRVEC')
  SubSystem1243 : Subsystem
  SubSystem1247 : Subsystem
  Block3380 : Outport
  wires:
  Block3366/1 -> Block3369/1
  Block3369/1 -> SubSystem1243/2(Block3373)
  Block3369/2 -> SubSystem1247/2(Block3377)
  SubSystem1243/1(Block3375) -> Block3370/1
  SubSystem1247/1(Block3379) -> Block3370/2
  Block3371/1 -> Block3380/1
  SubSystem1242/1(Output) -> Block3371/1
  Block3370/1 -> Block3371/2
  Block3367/1 -> SubSystem1247/1(Block3376), SubSystem1243/1(Block3372), SubSystem1242/1(element)

== system lib_all/SubSystem1196/SubSystem1206/SubSystem1208/SubSystem1217/SubSystem1251 ==
  Block3381 : Inport
  Block3382 : Inport  Port=2
  Block3383 : EnablePort
  Block3384 : Demux  Outputs=2
  Block3385 : Mux  Inputs=2
  Block3386 : Sum  OutDataTypeStr=double  RndMeth=Zero  SaturateOnIntegerOverflow=on  InputSameDT=on
  SubSystem1252 : Subsystem  TreatAsAtomicUnit=on  mask(***ATC.DSRVEC***){bd_version=1.01.A, r0=DSM3, r1=-1, r2=, r3=, r4=, r5=, r6=, r7=, r8=, r9=, c0=, c1=, c2=, c3=, c4=, c5=, c6=, c7=, c8=, c9=}
      mask prompts: bd_version "Version"
      mask help: これはAutoCからのブロックです．編集禁止．
      settings: OpenFcn=warndlg('変更禁止!');
      mask Help: web(ui_blockhelpmng(gcb));
      mask Display: disp('ATC.DSRVEC')
  SubSystem1253 : Subsystem
  SubSystem1257 : Subsystem
  Block3395 : Outport
  wires:
  Block3385/1 -> Block3386/2
  SubSystem1252/1(Output) -> Block3386/1
  Block3386/1 -> Block3395/1
  SubSystem1257/1(Block3394) -> Block3385/2
  SubSystem1253/1(Block3390) -> Block3385/1
  Block3384/2 -> SubSystem1257/2(Block3392)
  Block3384/1 -> SubSystem1253/2(Block3388)
  Block3381/1 -> Block3384/1
  Block3382/1 -> SubSystem1257/1(Block3391), SubSystem1253/1(Block3387), SubSystem1252/1(element)

== system lib_all/SubSystem1196/SubSystem1206/SubSystem1261/SubSystem1263/SubSystem1264 ==
  Block3410 : Inport
  Block3411 : Inport  Port=2
  Block3412 : Constant  Value=Parameter32  OutDataTypeStr=double  SampleTime=-1
  Block3413 : Constant  Value=2  OutDataTypeStr=double  SampleTime=-1
  Block3414 : Constant  Value=Parameter42  OutDataTypeStr=double  SampleTime=-1
  Block3415 : Constant  Value=0  OutDataTypeStr=double  SampleTime=-1
  Block3416 : Constant  Value=72  OutDataTypeStr=double  SampleTime=-1
  Block3417 : Constant  Value=Parameter34  OutDataTypeStr=double  SampleTime=-1
  Block3418 : Logic  Operator=OR
  Block3419 : Sum  Inputs=+-  OutDataTypeStr=double  RndMeth=Zero  SaturateOnIntegerOverflow=on  InputSameDT=on
  Block3420 : Switch  Threshold=0.5  RndMeth=Zero  SaturateOnIntegerOverflow=on  InputSameDT=on
  Block3421 : Switch  Threshold=0.5  RndMeth=Zero  SaturateOnIntegerOverflow=on  InputSameDT=on
  SubSystem1265 : Subsystem
  SubSystem1266 : Subsystem
  SubSystem1267 : Subsystem
      settings: RTWSystemCode=Inline
  Block3428 : Outport
  wires:
  Block3420/1 -> Block3419/2
  Block3417/1 -> Block3420/3
  Block3414/1 -> Block3420/1
  Block3418/1 -> Block3421/2
  SubSystem1266/1(Out1) -> Block3418/2
  SubSystem1265/1(Out1) -> Block3420/2, Block3418/1
  Block3413/1 -> SubSystem1266/2(In2)
  Block3412/1 -> SubSystem1265/1(In1)
  Block3411/1 -> SubSystem1266/1(In1), SubSystem1265/2(In2)
  Block3410/1 -> Block3419/1, Block3421/3
  Block3419/1 -> SubSystem1267/2(Block3423)
  Block3415/1 -> SubSystem1267/3(Block3424)
  Block3416/1 -> SubSystem1267/1(Block3422)
  SubSystem1267/1(Block3427) -> Block3421/1
  Block3421/1 -> Block3428/1
